simulink model "r_1f_2x_4a_r340"  (Simulink 7.2)
settings: Solver=ode45  StartTime=0.0  StopTime=1  RelTol=1e-3
undefined symbols (not defined in the file): ROACH
libraries: xbsIndex_r4 x2477, xps_library x100, casper_library x62, xbsReplacements_r4 x50
(part 3 of 20)

== system r_1f_2x_4a_r340/Subsystem4 ==
  led_up : Inport  OutDataType=sfix(16)  OutScaling=2^0
  led_rx : Inport  Port=2  OutDataType=sfix(16)  OutScaling=2^0
  data_in : Inport  Port=3  OutDataType=sfix(16)  OutScaling=2^0
  valid_in : Inport  Port=4  OutDataType=sfix(16)  OutScaling=2^0
  ip_in : Inport  Port=5  OutDataType=sfix(16)  OutScaling=2^0
  eof_in : Inport  Port=6  OutDataType=sfix(16)  OutScaling=2^0
  bad_frame : Inport  Port=7  OutDataType=sfix(16)  OutScaling=2^0
  overrun : Inport  Port=8  OutDataType=sfix(16)  OutScaling=2^0
  ack_in : Inport  Port=9  OutDataType=sfix(16)  OutScaling=2^0
  Concat1 : xbsIndex_r4/Concat  num_inputs=8  dbl_ovrd=off  block_type=concat
      settings: block_version=10.1.3
  Convert{1..6,18} (7 alike) : xbsIndex_r4/Convert  arith_type=Unsigned  n_bits=1  bin_pt=0  quantization=Truncate  overflow=Wrap  latency=0  dbl_ovrd=off  pipeline=off  block_type=convert
      settings: xl_area=[0, 0, 0, 0, 0, 0, 0]  block_version=10.1.3
  Delay{1..4} (4 alike) : xbsIndex_r4/Delay  en=off  latency=2  dbl_ovrd=off  reg_retiming=on  block_type=delay
      settings: xl_area=[0, 0, 0, 0, 0, 0, 0]
  Delay{5..22} (18 alike) : xbsIndex_r4/Delay  en=off  latency=1  dbl_ovrd=off  reg_retiming=on  block_type=delay
      settings: xl_area=[0, 0, 0, 0, 0, 0, 0]
  Logical4 : xbsIndex_r4/Logical  logical_function=AND  inputs=2  en=off  latency=0  precision=Full  arith_type=Unsigned  n_bits=8  bin_pt=2  align_bp=on  dbl_ovrd=off  block_type=logical
      settings: xl_area=[0, 0, 0, 0, 0, 0, 0]
  Slice11 : xbsIndex_r4/Slice  nbits=32-5  boolean_output=off  mode=Lower Bit Location + Width  bit1=0  base1=MSB of Input  bit0=0  base0=LSB of Input  dbl_ovrd=off  block_type=slice
      settings: infoedit="Extracts a given range of bits from each input sample and presents it at the output.  The output type is ordinarily unsigned with binary point at zero, but can be Boolean when the slice is one bit wide.<P><P>Hardware notes: In hardware this block costs nothing."  block_version=10.1.3
  data_out : Outport  OutDataType=sfix(16)  OutScaling=2^0
  oob_out : Outport  Port=2  OutDataType=sfix(16)  OutScaling=2^0
  trig_out : Outport  Port=3  OutDataType=sfix(16)  OutScaling=2^0
  we_out : Outport  Port=4  OutDataType=sfix(16)  OutScaling=2^0
  wires:
  Convert2/1 -> Concat1/6
  Convert1/1 -> Concat1/5
  Convert18/1 -> Concat1/4
  Convert4/1 -> Concat1/3
  Convert3/1 -> Concat1/2
  Slice11/1 -> Concat1/1
  Delay8/1 -> Delay17/1
  led_rx/1 -> Delay8/1
  Delay7/1 -> Delay16/1
  led_up/1 -> Delay7/1
  Delay11/1 -> Delay20/1
  overrun/1 -> Delay11/1
  Delay10/1 -> Delay19/1
  bad_frame/1 -> Delay10/1
  Delay13/1 -> Delay22/1
  Delay12/1 -> Delay21/1
  Delay9/1 -> Delay18/1
  Delay6/1 -> Delay15/1
  Delay5/1 -> Delay14/1
  Delay3/1 -> we_out/1
  Delay2/1 -> trig_out/1
  ack_in/1 -> Delay13/1
  eof_in/1 -> Delay9/1
  valid_in/1 -> Delay12/1
  Logical4/1 -> Delay2/1
  Delay4/1 -> oob_out/1
  Delay1/1 -> data_out/1
  ip_in/1 -> Delay6/1
  data_in/1 -> Delay5/1
  Concat1/1 -> Delay4/1
  Convert5/1 -> Concat1/7
  Convert6/1 -> Concat1/8
  Delay14/1 -> Delay1/1
  Delay15/1 -> Slice11/1
  Delay16/1 -> Convert3/1
  Delay17/1 -> Convert4/1
  Delay18/1 -> Convert18/1, Logical4/1
  Delay19/1 -> Convert1/1
  Delay20/1 -> Convert2/1
  Delay21/1 -> Convert5/1, Delay3/1, Logical4/2
  Delay22/1 -> Convert6/1

== system r_1f_2x_4a_r340/Subsystem5 ==
  rst : Inport  OutDataType=sfix(16)  OutScaling=2^0
  we : Inport  Port=2  OutDataType=sfix(16)  OutScaling=2^0
  eof : Inport  Port=3  OutDataType=sfix(16)  OutScaling=2^0
  flag : Inport  Port=4  OutDataType=sfix(16)  OutScaling=2^0
  Delay{1..3,40} (4 alike) : xbsIndex_r4/Delay  en=off  latency=2  dbl_ovrd=off  reg_retiming=on  block_type=delay
      settings: xl_area=[0, 0, 0, 0, 0, 0, 0]
  Logical2 : xbsIndex_r4/Logical  logical_function=AND  inputs=2  en=off  latency=0  precision=Full  arith_type=Unsigned  n_bits=8  bin_pt=2  align_bp=on  dbl_ovrd=off  block_type=logical
      settings: xl_area=[0, 0, 0, 0, 0, 0, 0]
  payload_cnt1 : xbsIndex_r4/Counter  cnt_type=Free Running  cnt_to=0  operation=Up  start_count=0  cnt_by_val=1  arith_type=Unsigned  n_bits=32  bin_pt=0  load_pin=off  rst=on  en=on  explicit_period=on  period=1  dbl_ovrd=off  use_behavioral_HDL=off  implementation=Fabric  use_rpm=on  block_type=counter
      settings: xl_area=[0, 0, 0, 0, 0, 0, 0]
  payload_cnt2 : xbsIndex_r4/Counter  cnt_type=Free Running  cnt_to=0  operation=Up  start_count=0  cnt_by_val=1  arith_type=Unsigned  n_bits=32  bin_pt=0  load_pin=off  rst=on  en=on  explicit_period=on  period=1  dbl_ovrd=off  use_behavioral_HDL=off  implementation=Fabric  use_rpm=on  block_type=counter
      settings: xl_area=[0, 0, 0, 0, 0, 0, 0]
  posedge : casper_library/Misc/posedge  Tag=Rising Edge Detector  SystemSampleTime=-1
  rx_cnt : Outport  OutDataType=sfix(16)  OutScaling=2^0
  err_cnt : Outport  Port=2  OutDataType=sfix(16)  OutScaling=2^0
  wires:
  Logical2/1 -> posedge/1
  posedge/1 -> payload_cnt1/2
  eof/1 -> Delay1/1
  rst/1 -> Delay2/1
  payload_cnt1/1 -> rx_cnt/1
  we/1 -> Delay40/1
  Delay40/1 -> Logical2/1
  Delay1/1 -> Logical2/2
  Delay2/1 -> payload_cnt1/1, payload_cnt2/1
  flag/1 -> Delay3/1
  payload_cnt2/1 -> err_cnt/1
  Delay3/1 -> payload_cnt2/2

== system r_1f_2x_4a_r340/Subsystem9 ==
  data_in : Inport  OutDataType=sfix(16)  OutScaling=2^0
  oob_in : Inport  Port=2  OutDataType=sfix(16)  OutScaling=2^0
  we_in : Inport  Port=3  OutDataType=sfix(16)  OutScaling=2^0
  link_down : Inport  Port=4  OutDataType=sfix(16)  OutScaling=2^0
  Concat2 : xbsIndex_r4/Concat  num_inputs=2  dbl_ovrd=off  block_type=concat
      settings: block_version=10.1.3
  Constant9 : xbsIndex_r4/Constant  arith_type=Boolean  const=1  n_bits=1  bin_pt=0  explicit_period=on  period=1  equ=P=C  opselect=C  inp2=PCIN>>17  opr=+  inp1=P  carry=CIN  dbl_ovrd=off  block_type=constant
  Convert : xbsIndex_r4/Convert  arith_type=Unsigned  n_bits=32  bin_pt=0  quantization=Truncate  overflow=Wrap  latency=0  dbl_ovrd=off  pipeline=off  block_type=convert
      settings: block_version=10.1.3
  Convert1 : xbsIndex_r4/Convert  arith_type=Unsigned  n_bits=1  bin_pt=0  quantization=Truncate  overflow=Wrap  latency=0  dbl_ovrd=off  pipeline=off  block_type=convert
      settings: block_version=10.1.3
  Delay{1..2,4,6} (4 alike) : xbsIndex_r4/Delay  en=off  latency=2  dbl_ovrd=off  reg_retiming=on  block_type=delay
      settings: xl_area=[0, 0, 0, 0, 0, 0, 0]
  Slice3 : xbsIndex_r4/Slice  nbits=1  boolean_output=on  mode=Lower Bit Location + Width  bit1=0  base1=MSB of Input  bit0=1  base0=LSB of Input  dbl_ovrd=off  block_type=slice
      settings: infoedit="Extracts a given range of bits from each input sample and presents it at the output.  The output type is ordinarily unsigned with binary point at zero, but can be Boolean when the slice is one bit wide.<P><P>Hardware notes: In hardware this block costs nothing."  block_version=10.1.3
  data_out : Outport  OutDataType=sfix(16)  OutScaling=2^0
  oob_out : Outport  Port=2  OutDataType=sfix(16)  OutScaling=2^0
  we_out : Outport  Port=3  OutDataType=sfix(16)  OutScaling=2^0
  trig_out : Outport  Port=4  OutDataType=sfix(16)  OutScaling=2^0
  stop : Outport  Port=5  OutDataType=sfix(16)  OutScaling=2^0
  wires:
  Delay1/1 -> data_out/1
  Delay4/1 -> Convert/1
  Delay6/1 -> trig_out/1
  oob_in/1 -> Concat2/2, Slice3/1
  Slice3/1 -> Delay6/1
  Delay2/1 -> we_out/1
  we_in/1 -> Delay2/1
  link_down/1 -> Convert1/1
  data_in/1 -> Delay1/1
  Concat2/1 -> Delay4/1
  Convert/1 -> oob_out/1
  Constant9/1 -> stop/1
  Convert1/1 -> Concat2/1

== system r_1f_2x_4a_r340/demux_gbe0 ==
  we : Inport  OutDataType=sfix(16)  OutScaling=2^0
  mcnt : Inport  Port=2  OutDataType=sfix(16)  OutScaling=2^0
  Constant1 : xbsIndex_r4/Constant  arith_type=Unsigned  const=nx  n_bits=share_bits  bin_pt=0  explicit_period=on  period=1  equ=P=C  opselect=C  inp2=PCIN>>17  opr=+  inp1=P  carry=CIN  dbl_ovrd=off  block_type=constant
  Logical3 : xbsIndex_r4/Logical  logical_function=AND  inputs=2  en=off  latency=0  precision=Full  arith_type=Unsigned  n_bits=8  bin_pt=2  align_bp=on  dbl_ovrd=off  block_type=logical
      settings: xl_area=[0, 0, 0, 0, 0, 0, 0]
  Relational : xbsIndex_r4/Relational  mode=a=b  en=off  latency=0  dbl_ovrd=off  block_type=relational
      settings: xl_area=[0, 0, 0, 0, 0, 0, 0]  block_version=10.1.3
  Slice4 : xbsIndex_r4/Slice  nbits=share_bits  boolean_output=off  mode=Lower Bit Location + Width  bit1=0  base1=MSB of Input  bit0=n_xeng_bits-share_bits  base0=LSB of Input  dbl_ovrd=off  block_type=slice
      settings: infoedit="Extracts a given range of bits from each input sample and presents it at the output.  The output type is ordinarily unsigned with binary point at zero, but can be Boolean when the slice is one bit wide.<P><P>Hardware notes: In hardware this block costs nothing."  block_version=10.1.3
  valid : Outport  OutDataType=sfix(16)  OutScaling=2^0
  wires:
  we/1 -> Logical3/1
  Logical3/1 -> valid/1
  mcnt/1 -> Slice4/1
  Slice4/1 -> Relational/1
  Constant1/1 -> Relational/2
  Relational/1 -> Logical3/2

== system r_1f_2x_4a_r340/demux_gbe1 ==
  we : Inport  OutDataType=sfix(16)  OutScaling=2^0
  mcnt : Inport  Port=2  OutDataType=sfix(16)  OutScaling=2^0
  Constant1 : xbsIndex_r4/Constant  arith_type=Unsigned  const=nx  n_bits=share_bits  bin_pt=0  explicit_period=on  period=1  equ=P=C  opselect=C  inp2=PCIN>>17  opr=+  inp1=P  carry=CIN  dbl_ovrd=off  block_type=constant
  Logical3 : xbsIndex_r4/Logical  logical_function=AND  inputs=2  en=off  latency=0  precision=Full  arith_type=Unsigned  n_bits=8  bin_pt=2  align_bp=on  dbl_ovrd=off  block_type=logical
      settings: xl_area=[0, 0, 0, 0, 0, 0, 0]
  Relational : xbsIndex_r4/Relational  mode=a=b  en=off  latency=0  dbl_ovrd=off  block_type=relational
      settings: xl_area=[0, 0, 0, 0, 0, 0, 0]  block_version=10.1.3
  Slice4 : xbsIndex_r4/Slice  nbits=share_bits  boolean_output=off  mode=Lower Bit Location + Width  bit1=0  base1=MSB of Input  bit0=n_xeng_bits-share_bits  base0=LSB of Input  dbl_ovrd=off  block_type=slice
      settings: infoedit="Extracts a given range of bits from each input sample and presents it at the output.  The output type is ordinarily unsigned with binary point at zero, but can be Boolean when the slice is one bit wide.<P><P>Hardware notes: In hardware this block costs nothing."  block_version=10.1.3
  valid : Outport  OutDataType=sfix(16)  OutScaling=2^0
  wires:
  Relational/1 -> Logical3/2
  Constant1/1 -> Relational/2
  Slice4/1 -> Relational/1
  mcnt/1 -> Slice4/1
  Logical3/1 -> valid/1
  we/1 -> Logical3/1

== system r_1f_2x_4a_r340/gbe_err ==
  rst : Inport  OutDataType=sfix(16)  OutScaling=2^0
  discard : Inport  Port=2  OutDataType=sfix(16)  OutScaling=2^0
  eof : Inport  Port=3  OutDataType=sfix(16)  OutScaling=2^0
  valid : Inport  Port=4  OutDataType=sfix(16)  OutScaling=2^0
  Constant2 : xbsIndex_r4/Constant  arith_type=Unsigned  const=packet_len-1  n_bits=packet_bits+1  bin_pt=0  explicit_period=on  period=1  equ=P=C  opselect=C  inp2=PCIN>>17  opr=+  inp1=P  carry=CIN  dbl_ovrd=off  block_type=constant
  Delay{1..3,17} (4 alike) : xbsIndex_r4/Delay  en=off  latency=2  dbl_ovrd=off  reg_retiming=on  block_type=delay
      settings: xl_area=[0, 0, 0, 0, 0, 0, 0]
  Inverter3 : xbsIndex_r4/Inverter  en=off  latency=0  dbl_ovrd=off  block_type=inv
      settings: xl_area=[0, 0, 0, 0, 0, 0, 0]
  Logical1 : xbsIndex_r4/Logical  logical_function=AND  inputs=2  en=off  latency=0  precision=Full  arith_type=Unsigned  n_bits=8  bin_pt=2  align_bp=on  dbl_ovrd=off  block_type=logical
      settings: xl_area=[0, 0, 0, 0, 0, 0, 0]
  Logical2 : xbsIndex_r4/Logical  logical_function=OR  inputs=2  en=off  latency=0  precision=Full  arith_type=Unsigned  n_bits=8  bin_pt=2  align_bp=on  dbl_ovrd=off  block_type=logical
      settings: xl_area=[0, 0, 0, 0, 0, 0, 0]
  Logical3 : xbsIndex_r4/Logical  logical_function=AND  inputs=3  en=off  latency=0  precision=Full  arith_type=Unsigned  n_bits=8  bin_pt=2  align_bp=on  dbl_ovrd=off  block_type=logical
      settings: xl_area=[0, 0, 0, 0, 0, 0, 0]
  Logical4 : xbsIndex_r4/Logical  logical_function=AND  inputs=2  en=off  latency=0  precision=Full  arith_type=Unsigned  n_bits=8  bin_pt=2  align_bp=on  dbl_ovrd=off  block_type=logical
      settings: xl_area=[0, 0, 0, 0, 0, 0, 0]
  Logical5 : xbsIndex_r4/Logical  logical_function=AND  inputs=2  en=off  latency=0  precision=Full  arith_type=Unsigned  n_bits=8  bin_pt=2  align_bp=on  dbl_ovrd=off  block_type=logical
      settings: xl_area=[0, 0, 0, 0, 0, 0, 0]
  Logical6 : xbsIndex_r4/Logical  logical_function=OR  inputs=2  en=off  latency=0  precision=Full  arith_type=Unsigned  n_bits=8  bin_pt=2  align_bp=on  dbl_ovrd=off  block_type=logical
      settings: xl_area=[0, 0, 0, 0, 0, 0, 0]
  Relational1 : xbsIndex_r4/Relational  mode=a!=b  en=off  latency=0  dbl_ovrd=off  block_type=relational
      settings: xl_area=[0, 0, 0, 0, 0, 0, 0]  block_version=10.1.3
  payload_cnt : xbsIndex_r4/Counter  cnt_type=Free Running  cnt_to=0  operation=Up  start_count=0  cnt_by_val=1  arith_type=Unsigned  n_bits=32  bin_pt=0  load_pin=off  rst=on  en=on  explicit_period=off  period=1  dbl_ovrd=off  use_behavioral_HDL=off  implementation=Fabric  use_rpm=on  block_type=counter
      settings: xl_area=[0, 0, 0, 0, 0, 0, 0]
  payload_cnt1 : xbsIndex_r4/Counter  cnt_type=Free Running  cnt_to=0  operation=Up  start_count=0  cnt_by_val=1  arith_type=Unsigned  n_bits=32  bin_pt=0  load_pin=off  rst=on  en=on  explicit_period=off  period=1  dbl_ovrd=off  use_behavioral_HDL=off  implementation=Fabric  use_rpm=on  block_type=counter
      settings: xl_area=[0, 0, 0, 0, 0, 0, 0]
  payload_cnt2 : xbsIndex_r4/Counter  cnt_type=Free Running  cnt_to=packet_len - 1  operation=Up  start_count=0  cnt_by_val=1  arith_type=Unsigned  n_bits=packet_bits+1  bin_pt=0  load_pin=off  rst=on  en=on  explicit_period=off  period=1  dbl_ovrd=off  use_behavioral_HDL=off  implementation=Fabric  use_rpm=on  block_type=counter
      settings: xl_area=[0, 0, 0, 0, 0, 0, 0]
  tx_cnt : Outport  OutDataType=sfix(16)  OutScaling=2^0
  err_cnt : Outport  Port=2  OutDataType=sfix(16)  OutScaling=2^0
  wires:
  Inverter3/1 -> Logical3/1
  Logical3/1 -> payload_cnt/2
  discard/1 -> Delay1/1
  eof/1 -> Delay2/1
  valid/1 -> Delay3/1
  rst/1 -> Delay17/1
  payload_cnt/1 -> tx_cnt/1
  payload_cnt1/1 -> err_cnt/1
  Delay17/1 -> payload_cnt/1, payload_cnt1/1
  Delay1/1 -> Inverter3/1, Logical4/2, Logical6/1
  Delay2/1 -> Logical3/2, Logical6/2
  Delay3/1 -> Logical3/3, Logical4/1, payload_cnt2/2, Logical1/2
  payload_cnt2/1 -> Relational1/1
  Constant2/1 -> Relational1/2
  Relational1/1 -> Logical5/2
  Logical1/1 -> payload_cnt2/1, Logical5/1
  Logical5/1 -> Logical2/2
  Logical2/1 -> payload_cnt1/2
  Logical4/1 -> Logical2/1
  Logical6/1 -> Logical1/1

== system r_1f_2x_4a_r340/loopback_fifo0 ==
  note: Only take packets transmitted
      | to my own IP address
  din : Inport  OutDataType=sfix(16)  OutScaling=2^0
  we : Inport  Port=2  OutDataType=sfix(16)  OutScaling=2^0
  dest_ip : Inport  Port=3  OutDataType=sfix(16)  OutScaling=2^0
  eof : Inport  Port=4  OutDataType=sfix(16)  OutScaling=2^0
  discard : Inport  Port=5  OutDataType=sfix(16)  OutScaling=2^0
  my_ip : Inport  Port=6  OutDataType=sfix(16)  OutScaling=2^0
  re : Inport  Port=7  OutDataType=sfix(16)  OutScaling=2^0
  Concat : xbsIndex_r4/Concat  num_inputs=2  dbl_ovrd=off  block_type=concat
      settings: block_version=10.1.3
  Constant1 : xbsIndex_r4/Constant  arith_type=Boolean  const=1  n_bits=2  bin_pt=0  explicit_period=on  period=1  equ=P=C  opselect=C  inp2=PCIN>>17  opr=+  inp1=P  carry=CIN  dbl_ovrd=off  block_type=constant
  Convert : xbsIndex_r4/Convert  arith_type=Unsigned  n_bits=1  bin_pt=0  quantization=Truncate  overflow=Wrap  latency=0  dbl_ovrd=off  pipeline=off  block_type=convert
      settings: xl_area=[0, 0, 0, 0, 0, 0, 0]  block_version=10.1.3
  Inverter1 : xbsIndex_r4/Inverter  en=off  latency=0  dbl_ovrd=off  block_type=inv
      settings: xl_area=[0, 0, 0, 0, 0, 0, 0]
  Logical1 : xbsIndex_r4/Logical  logical_function=OR  inputs=2  en=off  latency=0  precision=Full  arith_type=Unsigned  n_bits=8  bin_pt=2  align_bp=on  dbl_ovrd=off  block_type=logical
      settings: xl_area=[0, 0, 0, 0, 0, 0, 0]
  Logical2 : xbsIndex_r4/Logical  logical_function=AND  inputs=2  en=off  latency=0  precision=Full  arith_type=Unsigned  n_bits=8  bin_pt=2  align_bp=on  dbl_ovrd=off  block_type=logical
      settings: xl_area=[0, 0, 0, 0, 0, 0, 0]
  Logical4 : xbsIndex_r4/Logical  logical_function=AND  inputs=2  en=off  latency=0  precision=Full  arith_type=Unsigned  n_bits=8  bin_pt=2  align_bp=on  dbl_ovrd=off  block_type=logical
      settings: xl_area=[0, 0, 0, 0, 0, 0, 0]
  Relational1 : xbsIndex_r4/Relational  mode=a=b  en=off  latency=1  dbl_ovrd=off  block_type=relational
      settings: xl_area=[0, 0, 0, 0, 0, 0, 0]  block_version=10.1.3
  Slice : xbsIndex_r4/Slice  nbits=1  boolean_output=on  mode=Upper Bit Location + Width  bit1=0  base1=MSB of Input  bit0=0  base0=LSB of Input  dbl_ovrd=off  block_type=slice
      settings: infoedit="Extracts a given range of bits from each input sample and presents it at the output.  The output type is ordinarily unsigned with binary point at zero, but can be Boolean when the slice is one bit wide.<P><P>Hardware notes: In hardware this block costs nothing."  block_version=10.1.3
  Slice1 : xbsIndex_r4/Slice  nbits=64  boolean_output=off  mode=Lower Bit Location + Width  bit1=-1  base1=MSB of Input  bit0=0  base0=LSB of Input  dbl_ovrd=off  block_type=slice
      settings: infoedit="Extracts a given range of bits from each input sample and presents it at the output.  The output type is ordinarily unsigned with binary point at zero, but can be Boolean when the slice is one bit wide.<P><P>Hardware notes: In hardware this block costs nothing."  block_version=10.1.3
  Terminator{1..4} (4 alike) : Terminator
  fifo : Subsystem
  rollback_fifo : Subsystem  mask{depth_bits=8}
      mask prompts: depth_bits "Fifo Depth (2^?):"
      mask help: A rollback fifo behaves as a normal fifo, except that data which are written in have to be "committed" before they become available for readout. Moreover, any data which are written in and have not been committed can be "discarded", and will never be made available for readout.
  dout : Outport  OutDataType=sfix(16)  OutScaling=2^0
  valid : Outport  Port=2  OutDataType=sfix(16)  OutScaling=2^0
  ip_addr : Outport  Port=3  OutDataType=sfix(16)  OutScaling=2^0
  eof_out : Outport  Port=4  OutDataType=sfix(16)  OutScaling=2^0
  data_rdy : Outport  Port=5  OutDataType=sfix(16)  OutScaling=2^0
  overflow : Outport  Port=6  OutDataType=sfix(16)  OutScaling=2^0
  wires:
  Logical2/1 -> Logical1/1
  Inverter1/1 -> Logical2/2
  discard/1 -> Logical1/2
  Logical1/1 -> rollback_fifo/4(discard)
  Convert/1 -> Concat/1
  din/1 -> Concat/2
  Concat/1 -> rollback_fifo/1(din)
  Logical4/1 -> rollback_fifo/3(commit)
  we/1 -> rollback_fifo/2(we)
  eof/1 -> Logical2/1, Logical4/1, Convert/1
  Relational1/1 -> Inverter1/1, Logical4/2
  dest_ip/1 -> Relational1/1
  Constant1/1 -> rollback_fifo/5(re)
  rollback_fifo/2(valid) -> fifo/2(valid_in)
  re/1 -> fifo/4(re)
  fifo/2(valid) -> valid/1
  fifo/3(eof) -> eof_out/1
  fifo/1(data) -> dout/1
  fifo/4(data_rdy) -> data_rdy/1
  my_ip/1 -> Relational1/2, ip_addr/1
  Slice1/1 -> fifo/1(d_in)
  rollback_fifo/1(dout) -> Slice1/1, Slice/1
  Slice/1 -> fifo/3(eof_in)
  rollback_fifo/5(empty) -> Terminator2/1
  rollback_fifo/4(full) -> Terminator1/1
  rollback_fifo/3(wr_ack) -> Terminator3/1
  fifo/5(we) -> Terminator4/1
  fifo/6(full) -> overflow/1

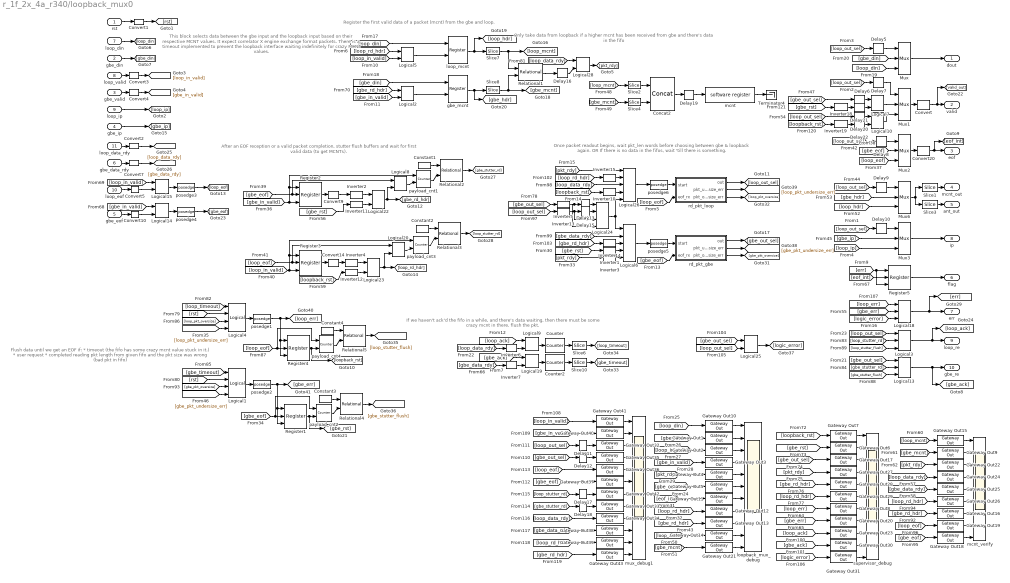
== system r_1f_2x_4a_r340/loopback_mux0 ==
  note: Once packet readout begins, wait pkt_len words
      | before choosing between gbe & loopback again.
      | OR
      | If there is no data in the fifos, wait 'till there is something.
  note: Only take data from loopback
      | if a higher mcnt has been
      | received from gbe and there's data
      | in the fifo
  note: This block selects data between the gbe input
      | and the loopback input based on their respective MCNT values.
      | It expect correlator X engine exchange format packets.
      |
      | There is a timeout implemented to prevent the loopback interface
      | waiting indefinitely for crazy MCNT values.
  note: Register the first valid
      | data of a packet (mcnt)
      | from the gbe and loop.
  note: After an EOF reception or a valid packet completion,
      |  stutter flush buffers and wait for first valid data (to get MCNTs).
  note: Flush data until we get an EOF if:
      |  * timeout (the fifo has some crazy mcnt value stuck in it.)
      |  * user request
      |  * completed reading pkt length from given fifo
      |     and the pkt size was wrong (bad pkt in fifo)
  note: If we haven't ack'd the fifo in a while,
      | and there's data waiting,
      | then there must be some crazy mcnt in there.
      | flush the pkt.
  rst : Inport  OutDataType=sfix(16)  OutScaling=2^0
  gbe_din : Inport  Port=2  OutDataType=sfix(16)  OutScaling=2^0
  gbe_valid : Inport  Port=3  OutDataType=sfix(16)  OutScaling=2^0
  gbe_ip : Inport  Port=4  OutDataType=sfix(16)  OutScaling=2^0
  gbe_eof : Inport  Port=5  OutDataType=sfix(16)  OutScaling=2^0
  gbe_data_rdy : Inport  Port=6  OutDataType=sfix(16)  OutScaling=2^0
  loop_din : Inport  Port=7  OutDataType=sfix(16)  OutScaling=2^0
  loop_valid : Inport  Port=8  OutDataType=sfix(16)  OutScaling=2^0
  loop_ip : Inport  Port=9  OutDataType=sfix(16)  OutScaling=2^0
  loop_eof : Inport  Port=10  OutDataType=sfix(16)  OutScaling=2^0
  loop_data_rdy : Inport  Port=11  OutDataType=sfix(16)  OutScaling=2^0
  Concat2 : xbsIndex_r4/Concat  num_inputs=2  dbl_ovrd=off  block_type=concat
      settings: block_version=10.1.3
  Constant{1..4} (4 alike) : xbsIndex_r4/Constant  arith_type=Unsigned  const=1  n_bits=ceil(log2(fifo_latency))  bin_pt=0  explicit_period=on  period=1  equ=P=C  opselect=C  inp2=PCIN>>17  opr=+  inp1=P  carry=CIN  dbl_ovrd=off  block_type=constant
  Convert{,1,9,14,20} (5 alike) : xbsIndex_r4/Convert  arith_type=Boolean  n_bits=8  bin_pt=6  quantization=Truncate  overflow=Wrap  latency=0  dbl_ovrd=off  pipeline=off  block_type=convert
      settings: xl_area=[0, 0, 0, 0, 0, 0, 0]  block_version=10.1.3
  Convert{2..5,7,10} (6 alike) : xbsIndex_r4/Convert  arith_type=Boolean  n_bits=1  bin_pt=0  quantization=Truncate  overflow=Wrap  latency=0  dbl_ovrd=off  pipeline=off  block_type=convert
      settings: xl_area=[0, 0, 0, 0, 0, 0, 0]  block_version=10.1.3
  Counter : xbsIndex_r4/Counter  cnt_type=Free Running  cnt_to=Inf  operation=Up  start_count=0  cnt_by_val=1  arith_type=Unsigned  n_bits=timeout_bits+1  bin_pt=0  load_pin=off  rst=on  en=off  explicit_period=off  period=1  dbl_ovrd=off  use_behavioral_HDL=off  implementation=Fabric  use_rpm=on  block_type=counter
      settings: xl_area=[0, 0, 0, 0, 0, 0, 0]
  Counter2 : xbsIndex_r4/Counter  cnt_type=Free Running  cnt_to=Inf  operation=Up  start_count=0  cnt_by_val=1  arith_type=Unsigned  n_bits=timeout_bits+1  bin_pt=0  load_pin=off  rst=on  en=off  explicit_period=off  period=1  dbl_ovrd=off  use_behavioral_HDL=off  implementation=Fabric  use_rpm=on  block_type=counter
      settings: xl_area=[0, 0, 0, 0, 0, 0, 0]
  Delay{5..13,15,17..18,20..22} (15 alike) : xbsIndex_r4/Delay  en=off  latency=fifo_latency  dbl_ovrd=off  reg_retiming=on  block_type=delay
      settings: xl_area=[0, 0, 0, 0, 0, 0, 0]
  Delay16 : xbsIndex_r4/Delay  en=off  latency=1  dbl_ovrd=off  reg_retiming=on  block_type=delay
      settings: xl_area=[0, 0, 0, 0, 0, 0, 0]
  Delay19 : xbsIndex_r4/Delay  en=off  latency=2  dbl_ovrd=off  reg_retiming=on  block_type=delay
      settings: xl_area=[0, 0, 0, 0, 0, 0, 0]
  Gateway Out{1..43} (43 alike) : xbsIndex_r4/Gateway Out  hdl_port=off  timing_constraint=None  locs_specified=off  LOCs={}  block_type=gatewayout
      settings: infoedit="Gateway out block.  Converts Xilinx fixed point inputs into ouputs of type Simulink integer, double, or fixed point.<P><P>Hardware notes:  In hardware these blocks become top level output ports or are discarded, depending on how they are configured."  xl_area=[0, 0, 0, 0, 0, 0, 0]  block_version=10.1.3
  Inverter{1..7,10..15,18..19} (15 alike) : xbsIndex_r4/Inverter  en=off  latency=0  dbl_ovrd=off  block_type=inv
      settings: xl_area=[0, 0, 0, 0, 0, 0, 0]
  Logical1 : xbsIndex_r4/Logical  logical_function=OR  inputs=4  en=off  latency=0  precision=Full  arith_type=Unsigned  n_bits=8  bin_pt=2  align_bp=on  dbl_ovrd=off  block_type=logical
      settings: xl_area=[0, 0, 0, 0, 0, 0, 0]
  Logical{2,5,7,10,14..15,22..23,25,28} (10 alike) : xbsIndex_r4/Logical  logical_function=AND  inputs=2  en=off  latency=0  precision=Full  arith_type=Unsigned  n_bits=8  bin_pt=2  align_bp=on  dbl_ovrd=off  block_type=logical
      settings: xl_area=[0, 0, 0, 0, 0, 0, 0]
  Logical13 : xbsIndex_r4/Logical  logical_function=OR  inputs=3  en=off  latency=0  precision=Full  arith_type=Unsigned  n_bits=8  bin_pt=2  align_bp=on  dbl_ovrd=off  block_type=logical
      settings: xl_area=[0, 0, 0, 0, 0, 0, 0]
  Logical18 : xbsIndex_r4/Logical  logical_function=OR  inputs=3  en=off  latency=1  precision=Full  arith_type=Unsigned  n_bits=8  bin_pt=2  align_bp=on  dbl_ovrd=off  block_type=logical
      settings: xl_area=[0, 0, 0, 0, 0, 0, 0]
  Logical{8..9,19..20} (4 alike) : xbsIndex_r4/Logical  logical_function=OR  inputs=2  en=off  latency=0  precision=Full  arith_type=Unsigned  n_bits=8  bin_pt=2  align_bp=on  dbl_ovrd=off  block_type=logical
      settings: xl_area=[0, 0, 0, 0, 0, 0, 0]
  Logical24 : xbsIndex_r4/Logical  logical_function=AND  inputs=4  en=off  latency=0  precision=Full  arith_type=Unsigned  n_bits=8  bin_pt=2  align_bp=on  dbl_ovrd=off  block_type=logical
      settings: xl_area=[0, 0, 0, 0, 0, 0, 0]
  Logical26 : xbsIndex_r4/Logical  logical_function=AND  inputs=5  en=off  latency=0  precision=Full  arith_type=Unsigned  n_bits=8  bin_pt=2  align_bp=on  dbl_ovrd=off  block_type=logical
      settings: xl_area=[0, 0, 0, 0, 0, 0, 0]
  Logical3 : xbsIndex_r4/Logical  logical_function=OR  inputs=3  en=off  latency=0  precision=Full  arith_type=Unsigned  n_bits=8  bin_pt=2  align_bp=on  dbl_ovrd=off  block_type=logical
      settings: xl_area=[0, 0, 0, 0, 0, 0, 0]
  Logical4 : xbsIndex_r4/Logical  logical_function=OR  inputs=4  en=off  latency=0  precision=Full  arith_type=Unsigned  n_bits=8  bin_pt=2  align_bp=on  dbl_ovrd=off  block_type=logical
      settings: xl_area=[0, 0, 0, 0, 0, 0, 0]
  Logical6 : xbsIndex_r4/Logical  logical_function=AND  inputs=5  en=off  latency=0  precision=Full  arith_type=Unsigned  n_bits=8  bin_pt=2  align_bp=on  dbl_ovrd=off  block_type=logical
      settings: xl_area=[0, 0, 0, 0, 0, 0, 0]
  Mux{,1..3,6} (5 alike) : xbsIndex_r4/Mux  inputs=2  en=off  latency=0  precision=Full  arith_type="Signed  (2's comp)"  n_bits=8  bin_pt=2  quantization=Truncate  overflow=Wrap  dbl_ovrd=off  block_type=mux
      settings: xl_area=[0, 0, 0, 0, 0, 0, 0]
  Register{1..5} (5 alike) : xbsIndex_r4/Register  init=0  rst=on  en=on  dbl_ovrd=off  block_type=register
      settings: xl_area=[0, 0, 0, 0, 0, 0, 0]
  Relational1 : xbsIndex_r4/Relational  mode=a<b  en=off  latency=1  dbl_ovrd=off  block_type=relational
      settings: xl_area=[0, 0, 0, 0, 0, 0, 0]  block_version=10.1.3
  Relational{2..5} (4 alike) : xbsIndex_r4/Relational  mode=a=b  en=off  latency=0  dbl_ovrd=off  block_type=relational
      settings: xl_area=[0, 0, 0, 0, 0, 0, 0]  block_version=10.1.3
  Slice1 : xbsIndex_r4/Slice  nbits=48  boolean_output=off  mode=Lower Bit Location + Width  bit1=0  base1=MSB of Input  bit0=16  base0=LSB of Input  dbl_ovrd=off  block_type=slice
      settings: infoedit="Extracts a given range of bits from each input sample and presents it at the output.  The output type is ordinarily unsigned with binary point at zero, but can be Boolean when the slice is one bit wide.<P><P>Hardware notes: In hardware this block costs nothing."  block_version=10.1.3
  Slice10 : xbsIndex_r4/Slice  nbits=1  boolean_output=on  mode=Upper Bit Location + Width  bit1=0  base1=MSB of Input  bit0=1  base0=LSB of Input  dbl_ovrd=off  block_type=slice
      settings: infoedit="Extracts a given range of bits from each input sample and presents it at the output.  The output type is ordinarily unsigned with binary point at zero, but can be Boolean when the slice is one bit wide.<P><P>Hardware notes: In hardware this block costs nothing."  block_version=10.1.3
  Slice2 : xbsIndex_r4/Slice  nbits=16  boolean_output=off  mode=Lower Bit Location + Width  bit1=0  base1=MSB of Input  bit0=0  base0=LSB of Input  dbl_ovrd=off  block_type=slice
      settings: infoedit="Extracts a given range of bits from each input sample and presents it at the output.  The output type is ordinarily unsigned with binary point at zero, but can be Boolean when the slice is one bit wide.<P><P>Hardware notes: In hardware this block costs nothing."  block_version=10.1.3
  Slice3 : xbsIndex_r4/Slice  nbits=16  boolean_output=off  mode=Lower Bit Location + Width  bit1=0  base1=MSB of Input  bit0=0  base0=LSB of Input  dbl_ovrd=off  block_type=slice
      settings: infoedit="Extracts a given range of bits from each input sample and presents it at the output.  The output type is ordinarily unsigned with binary point at zero, but can be Boolean when the slice is one bit wide.<P><P>Hardware notes: In hardware this block costs nothing."  block_version=10.1.3
  Slice4 : xbsIndex_r4/Slice  nbits=16  boolean_output=off  mode=Lower Bit Location + Width  bit1=0  base1=MSB of Input  bit0=0  base0=LSB of Input  dbl_ovrd=off  block_type=slice
      settings: infoedit="Extracts a given range of bits from each input sample and presents it at the output.  The output type is ordinarily unsigned with binary point at zero, but can be Boolean when the slice is one bit wide.<P><P>Hardware notes: In hardware this block costs nothing."  block_version=10.1.3
  Slice6 : xbsIndex_r4/Slice  nbits=1  boolean_output=on  mode=Upper Bit Location + Width  bit1=0  base1=MSB of Input  bit0=1  base0=LSB of Input  dbl_ovrd=off  block_type=slice
      settings: infoedit="Extracts a given range of bits from each input sample and presents it at the output.  The output type is ordinarily unsigned with binary point at zero, but can be Boolean when the slice is one bit wide.<P><P>Hardware notes: In hardware this block costs nothing."  block_version=10.1.3
  Slice7 : xbsIndex_r4/Slice  nbits=48  boolean_output=off  mode=Upper Bit Location + Width  bit1=0  base1=MSB of Input  bit0=0  base0=LSB of Input  dbl_ovrd=off  block_type=slice
      settings: infoedit="Extracts a given range of bits from each input sample and presents it at the output.  The output type is ordinarily unsigned with binary point at zero, but can be Boolean when the slice is one bit wide.<P><P>Hardware notes: In hardware this block costs nothing."  block_version=10.1.3
  Slice8 : xbsIndex_r4/Slice  nbits=48  boolean_output=off  mode=Upper Bit Location + Width  bit1=0  base1=MSB of Input  bit0=0  base0=LSB of Input  dbl_ovrd=off  block_type=slice
      settings: infoedit="Extracts a given range of bits from each input sample and presents it at the output.  The output type is ordinarily unsigned with binary point at zero, but can be Boolean when the slice is one bit wide.<P><P>Hardware notes: In hardware this block costs nothing."  block_version=10.1.3
  Terminator4 : Terminator
  gbe_mcnt : xbsIndex_r4/Register  init=0  rst=off  en=on  dbl_ovrd=off  block_type=register
      settings: xl_area=[0, 0, 0, 0, 0, 0, 0]
  loop_mcnt : xbsIndex_r4/Register  init=0  rst=off  en=on  dbl_ovrd=off  block_type=register
      settings: xl_area=[0, 0, 0, 0, 0, 0, 0]
  loopback_mux_debug : Scope  NumInputPorts=11
      settings: YMin=300~190.95~0~0.95~0.95~-1~-1~-1~-5~-5~-5  YMax=525~211.05~1~1.05~1.05~1~1~1~5~5~5  SaveName=ScopeData7
  mcnt : xps_library/software register  Tag=xps:sw_reg  SystemSampleTime=-1  io_dir=To Processor  arith_type=Unsigned  bitwidth=32  bin_pt=0  sample_period=1  gw_name=r_1f_2x_4a_r339_loopback_mux0_mcnt_user_data_in
      link override convert: arith_type=Unsigned  n_bits=32  bin_pt=0
  mcnt_verify : Scope  NumInputPorts=9
      settings: YMin=300~190.95~0~-5~-5~-5~-5~-5~-5  YMax=525~211.05~1~5~5~5~5~5~5  SaveName=ScopeData2
  mux_debug1 : Scope  NumInputPorts=12
      settings: YMin=300~190.95~0~-5~-5~-5~-5~-5~-5~-5~-5~-5  YMax=525~211.05~1~5~5~5~5~5~5~5~5~5  SaveName=ScopeData3
  payload_cnt{1..4} (4 alike) : xbsIndex_r4/Counter  cnt_type=Count Limited  cnt_to=max((fifo_latency + 1),2)  operation=Up  start_count=0  cnt_by_val=1  arith_type=Unsigned  n_bits=ceil(log2(fifo_latency))+1  bin_pt=0  load_pin=off  rst=on  en=on  explicit_period=on  period=1  dbl_ovrd=off  use_behavioral_HDL=off  implementation=Fabric  use_rpm=on  block_type=counter
      settings: xl_area=[0, 0, 0, 0, 0, 0, 0]
  posedge{1..6} (6 alike) : casper_library/Misc/posedge  Tag=Rising Edge Detector  SystemSampleTime=-1
  rd_pkt_gbe : Subsystem
  rd_pkt_loop : Subsystem
  supervisor_debug : Scope  NumInputPorts=11
      settings: YMin=300~190.95~0~-5~-5~-5~-5~-5~-5~-5~-5  YMax=525~211.05~1~5~5~5~5~5~5~5~5  SaveName=ScopeData18
  dout : Outport  OutDataType=sfix(16)  OutScaling=2^0
  valid : Outport  Port=2  OutDataType=sfix(16)  OutScaling=2^0
  eof : Outport  Port=3  OutDataType=sfix(16)  OutScaling=2^0
  mcnt_out : Outport  Port=4  OutDataType=sfix(16)  OutScaling=2^0
  ant_out : Outport  Port=5  OutDataType=sfix(16)  OutScaling=2^0
  flag : Outport  Port=6  OutDataType=sfix(16)  OutScaling=2^0
  err : Outport  Port=7  OutDataType=sfix(16)  OutScaling=2^0
  ip : Outport  Port=8  OutDataType=sfix(16)  OutScaling=2^0
  loop_re : Outport  Port=9  OutDataType=sfix(16)  OutScaling=2^0
  gbe_re : Outport  Port=10  OutDataType=sfix(16)  OutScaling=2^0
  wires:
  Mux/1 -> dout/1
  Mux1/1 -> Convert/1
  Mux2/1 -> Convert20/1
  gbe_valid/1 -> Convert4/1
  loop_valid/1 -> Convert3/1
  Convert/1 -> valid/1, [valid_out]
  Slice2/1 -> Concat2/1
  Slice4/1 -> Concat2/2
  Concat2/1 -> Delay19/1
  Delay5/1 -> Mux/1
  [loop_out_sel] -> Delay5/1
  [loop_out_sel] -> Delay8/1
  Gateway Out2/1 -> loopback_mux_debug/3
  Gateway Out3/1 -> loopback_mux_debug/4
  Gateway Out4/1 -> loopback_mux_debug/5
  Gateway Out5/1 -> loopback_mux_debug/6
  Convert3/1 -> [loop_in_valid]
  Convert4/1 -> [gbe_in_valid]
  Relational1/1 -> Delay16/1
  loop_din/1 -> [loop_din]
  gbe_din/1 -> [gbe_din]
  [gbe_din] -> Gateway Out1/1
  [loop_din] -> Gateway Out10/1
  [loop_in_valid] -> Gateway Out2/1
  [gbe_in_valid] -> Gateway Out3/1
  [gbe_out_sel] -> Gateway Out5/1
  [pkt_rdy] -> Gateway Out4/1
  Gateway Out11/1 -> loopback_mux_debug/7
  [eof_int] -> Gateway Out11/1
  [loop_rd_hdr] -> Gateway Out12/1
  Gateway Out12/1 -> loopback_mux_debug/8
  loop_eof/1 -> Convert5/1
  gbe_eof/1 -> Convert10/1
  Gateway Out13/1 -> loopback_mux_debug/9
  [gbe_rd_hdr] -> Gateway Out13/1
  [loop_out_sel] -> Delay7/1
  loop_ip/1 -> [loop_ip]
  gbe_ip/1 -> [gbe_ip]
  Mux3/1 -> ip/1
  [loop_out_sel] -> Delay10/1
  [loop_mcnt] -> Slice2/1
  [gbe_mcnt] -> Slice4/1
  Gateway Out10/1 -> loopback_mux_debug/1
  Gateway Out1/1 -> loopback_mux_debug/2
  Gateway Out14/1 -> loopback_mux_debug/10
  Gateway Out21/1 -> loopback_mux_debug/11
  [loop_mcnt] -> Gateway Out14/1
  [gbe_mcnt] -> Gateway Out21/1
  Gateway Out22/1 -> mcnt_verify/3
  Gateway Out15/1 -> mcnt_verify/1
  Gateway Out9/1 -> mcnt_verify/2
  Gateway Out24/1 -> mcnt_verify/4
  Gateway Out25/1 -> mcnt_verify/5
  Gateway Out26/1 -> mcnt_verify/6
  Gateway Out16/1 -> mcnt_verify/7
  [loop_mcnt] -> Gateway Out15/1
  [gbe_mcnt] -> Gateway Out9/1
  [pkt_rdy] -> Gateway Out22/1
  Slice7/1 -> Relational1/1, [loop_mcnt]
  Slice8/1 -> Relational1/2, [gbe_mcnt]
  Slice1/1 -> mcnt_out/1
  Slice3/1 -> ant_out/1
  Mux6/1 -> Slice1/1, Slice3/1
  Counter2/1 -> Slice10/1
  Logical1/1 -> posedge2/1
  Logical14/1 -> posedge4/1
  Logical15/1 -> posedge3/1
  Convert5/1 -> Logical15/2
  Convert10/1 -> Logical14/2
  [gbe_in_valid] -> Logical14/1
  [loop_in_valid] -> Logical15/1
  Gateway Out17/1 -> supervisor_debug/3
  Gateway Out7/1 -> supervisor_debug/1
  Gateway Out6/1 -> supervisor_debug/2
  Gateway Out27/1 -> supervisor_debug/4
  Gateway Out28/1 -> supervisor_debug/5
  Gateway Out29/1 -> supervisor_debug/6
  Gateway Out8/1 -> supervisor_debug/7
  [loopback_rst] -> Gateway Out7/1
  [gbe_rst] -> Gateway Out6/1
  [gbe_out_sel] -> Gateway Out17/1
  [pkt_rdy] -> Gateway Out27/1
  [gbe_rd_hdr] -> Gateway Out28/1
  [loop_rd_hdr] -> Gateway Out29/1
  Logical3/1 -> loop_re/1, [loop_ack]
  Logical13/1 -> gbe_re/1, [gbe_ack]
  rst/1 -> Convert1/1
  Logical18/1 -> err/1, [err]
  Counter/1 -> Slice6/1
  Logical4/1 -> posedge1/1
  Register2/1 -> Convert9/1
  Logical22/1 -> [gbe_rd_hdr], Logical8/2
  Register3/1 -> Convert14/1
  Convert14/1 -> Inverter4/1
  gbe_mcnt/1 -> Slice8/1, [gbe_hdr]
  loop_mcnt/1 -> Slice7/1, [loop_hdr]
  Logical5/1 -> loop_mcnt/2
  [loop_rd_hdr] -> Logical5/1
  [gbe_rd_hdr] -> Logical2/1
  [loop_in_valid] -> Logical5/2
  [gbe_in_valid] -> Logical2/2
  [loop_din] -> loop_mcnt/1
  [gbe_din] -> gbe_mcnt/1
  Convert1/1 -> [rst]
  Convert20/1 -> [eof_int], eof/1
  posedge1/1 -> Register4/3, Register4/1, [loop_err]
  posedge2/1 -> Register1/1, Register1/3, [gbe_err]
  posedge3/1 -> [loop_eof]
  posedge4/1 -> [gbe_eof]
  Logical2/1 -> gbe_mcnt/2
  [loop_out_sel] -> Delay20/1
  Delay19/1 -> mcnt/1
  mcnt/1 -> Terminator4/1
  gbe_data_rdy/1 -> Convert7/1
  loop_data_rdy/1 -> Convert2/1
  Convert2/1 -> [loop_data_rdy]
  Convert7/1 -> [gbe_data_rdy]
  payload_cnt1/1 -> Relational2/2
  Constant1/1 -> Relational2/1
  payload_cnt3/1 -> Relational3/2
  Constant2/1 -> Relational3/1
  [gbe_out_sel] -> Delay6/1
  posedge6/1 -> rd_pkt_loop/1(start)
  rd_pkt_loop/1(out) -> [loop_out_sel]
  rd_pkt_gbe/1(out) -> [gbe_out_sel]
  [gbe_in_valid] -> Register2/3, Register2/1, payload_cnt1/1
  [loop_in_valid] -> Register3/3, Register3/1, payload_cnt3/1
  [loop_eof] -> Register3/2, Logical20/1
  [gbe_eof] -> Register2/2, Logical8/1
  Logical8/1 -> payload_cnt1/2
  Logical20/1 -> payload_cnt3/2
  Relational2/1 -> [gbe_stutter_rd]
  Relational3/1 -> [loop_stutter_rd]
  rd_pkt_gbe/3(pkt_oversize_err) -> [gbe_pkt_oversize]
  Slice6/1 -> [loop_timeout]
  Slice10/1 -> [gbe_timeout]
  [rst] -> Logical1/2
  [rst] -> Logical4/2
  [gbe_timeout] -> Logical1/1
  [loop_timeout] -> Logical4/1
  [gbe_pkt_oversize] -> Logical1/3
  [loop_pkt_oversize] -> Logical4/3
  [gbe_eof] -> Register1/2, payload_cnt2/1
  payload_cnt2/1 -> Relational4/2
  Constant3/1 -> Relational4/1
  Relational4/1 -> [gbe_stutter_flush]
  Register1/1 -> payload_cnt2/2, [gbe_rst]
  [loop_eof] -> payload_cnt4/1, Register4/2
  payload_cnt4/1 -> Relational5/2
  Constant4/1 -> Relational5/1
  Register4/1 -> payload_cnt4/2, [loopback_rst]
  Relational5/1 -> [loop_stutter_flush]
  [gbe_out_sel] -> Logical13/1
  [gbe_stutter_rd] -> Logical13/2
  [gbe_stutter_flush] -> Logical13/3
  [loop_out_sel] -> Logical3/1
  [loop_stutter_rd] -> Logical3/2
  [loop_stutter_flush] -> Logical3/3
  Inverter2/1 -> Logical22/1
  Convert9/1 -> Inverter2/1
  [gbe_rst] -> Inverter11/1
  Inverter11/1 -> Logical22/2
  Inverter12/1 -> Logical23/2
  Logical23/1 -> Logical20/2, [loop_rd_hdr]
  Inverter4/1 -> Logical23/1
  [loopback_rst] -> Inverter12/1
  Gateway Out19/1 -> mcnt_verify/8
  Gateway Out18/1 -> mcnt_verify/9
  [loop_data_rdy] -> Gateway Out24/1
  [gbe_data_rdy] -> Gateway Out25/1
  [loop_rd_hdr] -> Gateway Out26/1
  [gbe_rd_hdr] -> Gateway Out16/1
  [loop_eof] -> Gateway Out19/1
  [gbe_eof] -> Gateway Out18/1
  Gateway Out20/1 -> supervisor_debug/8
  Gateway Out23/1 -> supervisor_debug/9
  [loop_err] -> Gateway Out8/1
  [gbe_err] -> Gateway Out20/1
  Gateway Out30/1 -> supervisor_debug/10
  [gbe_out_sel] -> Inverter5/1
  [loop_out_sel] -> Inverter13/1
  Inverter5/1 -> Delay13/1, Logical24/4
  Inverter13/1 -> Delay15/1, Logical24/3
  [loop_ack] -> Gateway Out23/1
  [gbe_ack] -> Gateway Out30/1
  [loop_rd_hdr] -> Inverter15/1
  [gbe_rd_hdr] -> Inverter14/1
  Delay8/1 -> Mux2/1
  Delay9/1 -> Mux6/1
  [loop_out_sel] -> Delay9/1
  Delay10/1 -> Mux3/1
  Delay7/1 -> Mux1/1
  [gbe_out_sel] -> Logical25/1
  [loop_out_sel] -> Logical25/2
  Logical25/1 -> [logic_error]
  Gateway Out31/1 -> supervisor_debug/11
  [logic_error] -> Gateway Out31/1
  Gateway Out32/1 -> mux_debug1/3
  Gateway Out41/1 -> mux_debug1/1
  Gateway Out40/1 -> mux_debug1/2
  Gateway Out35/1 -> mux_debug1/4
  Gateway Out36/1 -> mux_debug1/5
  Gateway Out37/1 -> mux_debug1/6
  Gateway Out42/1 -> mux_debug1/7
  Gateway Out33/1 -> mux_debug1/8
  Gateway Out34/1 -> mux_debug1/9
  Gateway Out38/1 -> mux_debug1/10
  Gateway Out39/1 -> mux_debug1/11
  [loop_in_valid] -> Gateway Out41/1
  [gbe_in_valid] -> Gateway Out40/1
  [loop_eof] -> Gateway Out36/1
  [gbe_eof] -> Gateway Out37/1
  [gbe_out_sel] -> Delay12/1
  [loop_out_sel] -> Delay11/1
  [loop_stutter_rd] -> Delay17/1
  [gbe_stutter_rd] -> Delay18/1
  Delay11/1 -> Gateway Out32/1
  Delay12/1 -> Gateway Out35/1
  Delay17/1 -> Gateway Out42/1
  Delay18/1 -> Gateway Out33/1
  [loop_data_rdy] -> Gateway Out34/1
  [gbe_data_rdy] -> Gateway Out38/1
  Gateway Out43/1 -> mux_debug1/12
  [loop_rd_hdr] -> Gateway Out39/1
  [gbe_rd_hdr] -> Gateway Out43/1
  Delay13/1 -> Logical24/1
  Delay15/1 -> Logical24/2
  [loopback_rst] -> Inverter10/1
  [gbe_rst] -> Inverter1/1
  [gbe_eof] -> rd_pkt_gbe/2(eof_rx)
  [gbe_din] -> Mux/2
  [loop_din] -> Mux/3
  Logical10/1 -> Mux1/3
  Logical7/1 -> Mux1/2
  [gbe_eof] -> Mux2/2
  [loop_eof] -> Mux2/3
  [gbe_hdr] -> Mux6/2
  [loop_hdr] -> Mux6/3
  [gbe_ip] -> Mux3/2
  [loop_ip] -> Mux3/3
  Delay6/1 -> Logical7/1
  rd_pkt_gbe/2(pkt_undersize_err) -> [gbe_pkt_undersize_err]
  rd_pkt_loop/2(pkt_undersize_err) -> [loop_pkt_undersize_err]
  [loop_err] -> Logical18/1
  [gbe_err] -> Logical18/2
  Logical9/1 -> Counter/1
  [loop_ack] -> Logical9/1
  [loop_data_rdy] -> Inverter6/1
  Inverter6/1 -> Logical9/2
  Inverter7/1 -> Logical19/2
  Logical19/1 -> Counter2/1
  [gbe_ack] -> Logical19/1
  [gbe_data_rdy] -> Inverter7/1
  Logical6/1 -> posedge5/1
  [loop_data_rdy] -> Logical26/3
  [gbe_data_rdy] -> Logical6/2
  [loop_data_rdy] -> Logical28/1
  Delay16/1 -> Logical28/2
  Logical28/1 -> [pkt_rdy]
  Inverter14/1 -> Logical6/3
  Inverter10/1 -> Logical26/4
  Inverter1/1 -> Logical6/4
  Inverter15/1 -> Logical26/2
  posedge5/1 -> rd_pkt_gbe/1(start)
  [pkt_rdy] -> Inverter3/1
  [loopback_rst] -> Inverter19/1
  [gbe_rst] -> Inverter18/1
  Inverter18/1 -> Delay21/1
  Inverter19/1 -> Delay22/1
  [loop_pkt_undersize_err] -> Logical4/4
  [gbe_pkt_undersize_err] -> Logical1/4
  Delay21/1 -> Logical7/2
  [loop_eof] -> rd_pkt_loop/2(eof_rx)
  rd_pkt_loop/3(pkt_oversize_err) -> [loop_pkt_oversize]
  [logic_error] -> Logical18/3
  Logical24/1 -> Logical6/1, Logical26/5
  Delay20/1 -> Logical10/1
  Delay22/1 -> Logical10/2
  [pkt_rdy] -> Logical26/1
  Inverter3/1 -> Logical6/5
  Logical26/1 -> posedge6/1
  [err] -> Register5/1, Register5/3
  [eof_int] -> Register5/2
  Register5/1 -> flag/1

== system r_1f_2x_4a_r340/miss_cnt0 ==
  rst : Inport  OutDataType=sfix(16)  OutScaling=2^0
  valid : Inport  Port=2  OutDataType=sfix(16)  OutScaling=2^0
  rcvd : Inport  Port=3  OutDataType=sfix(16)  OutScaling=2^0
  sync : Inport  Port=4  OutDataType=sfix(16)  OutScaling=2^0
  Counter1 : xbsIndex_r4/Counter  cnt_type=Free Running  cnt_to=1023  operation=Up  start_count=0  cnt_by_val=1  arith_type=Unsigned  n_bits=32  bin_pt=0  load_pin=off  rst=on  en=on  explicit_period=on  period=1  dbl_ovrd=off  use_behavioral_HDL=off  implementation=Fabric  use_rpm=on  block_type=counter
      settings: xl_area=[0, 0, 0, 0, 0, 0, 0]
  Counter3 : xbsIndex_r4/Counter  cnt_type=Free Running  cnt_to=1023  operation=Up  start_count=0  cnt_by_val=1  arith_type=Unsigned  n_bits=32  bin_pt=0  load_pin=off  rst=on  en=on  explicit_period=on  period=1  dbl_ovrd=off  use_behavioral_HDL=off  implementation=Fabric  use_rpm=on  block_type=counter
      settings: xl_area=[0, 0, 0, 0, 0, 0, 0]
  Delay{1..3,55} (4 alike) : xbsIndex_r4/Delay  en=off  latency=2  dbl_ovrd=off  reg_retiming=on  block_type=delay
      settings: xl_area=[0, 0, 0, 0, 0, 0, 0]
  Inverter : xbsIndex_r4/Inverter  en=off  latency=0  dbl_ovrd=off  block_type=inv
      settings: xl_area=[0, 0, 0, 0, 0, 0, 0]
  Logical2 : xbsIndex_r4/Logical  logical_function=AND  inputs=2  en=off  latency=0  precision=Full  arith_type=Unsigned  n_bits=8  bin_pt=2  align_bp=on  dbl_ovrd=off  block_type=logical
      settings: xl_area=[0, 0, 0, 0, 0, 0, 0]
  Register : xbsIndex_r4/Register  init=0  rst=off  en=on  dbl_ovrd=off  block_type=register
  Slice : xbsIndex_r4/Slice  nbits=16  boolean_output=off  mode=Two Bit Locations  bit1=0  base1=MSB of Input  bit0=xeng_int_bits  base0=LSB of Input  dbl_ovrd=off  block_type=slice
      settings: infoedit="Extracts a given range of bits from each input sample and presents it at the output.  The output type is ordinarily unsigned with binary point at zero, but can be Boolean when the slice is one bit wide.<P><P>Hardware notes: In hardware this block costs nothing."  block_version=10.1.3
  ant_cnt : xbsIndex_r4/Counter  cnt_type=Free Running  cnt_to=1023  operation=Up  start_count=0  cnt_by_val=1  arith_type=Unsigned  n_bits=xeng_int_bits + n_ants_bits  bin_pt=0  load_pin=off  rst=on  en=on  explicit_period=on  period=1  dbl_ovrd=off  use_behavioral_HDL=off  implementation=Fabric  use_rpm=on  block_type=counter
      settings: xl_area=[0, 0, 0, 0, 0, 0, 0]
  spectr_cnt : Outport  OutDataType=sfix(16)  OutScaling=2^0
  err_cnt : Outport  Port=2  OutDataType=sfix(16)  OutScaling=2^0
  last_missed_ant : Outport  Port=3  OutDataType=sfix(16)  OutScaling=2^0
  wires:
  Logical2/1 -> Counter3/2, Register/2
  rst/1 -> Delay55/1
  valid/1 -> Delay1/1
  Counter3/1 -> err_cnt/1
  rcvd/1 -> Delay2/1
  Inverter/1 -> Logical2/2
  Delay55/1 -> Counter3/1, Counter1/1
  Delay1/1 -> Logical2/1, ant_cnt/2
  Delay2/1 -> Inverter/1
  Delay3/1 -> Counter1/2, ant_cnt/1
  Counter1/1 -> spectr_cnt/1
  sync/1 -> Delay3/1
  Register/1 -> last_missed_ant/1
  ant_cnt/1 -> Slice/1
  Slice/1 -> Register/1

== system r_1f_2x_4a_r340/miss_cnt1 ==
  rst : Inport  OutDataType=sfix(16)  OutScaling=2^0
  valid : Inport  Port=2  OutDataType=sfix(16)  OutScaling=2^0
  rcvd : Inport  Port=3  OutDataType=sfix(16)  OutScaling=2^0
  sync : Inport  Port=4  OutDataType=sfix(16)  OutScaling=2^0
  Counter1 : xbsIndex_r4/Counter  cnt_type=Free Running  cnt_to=1023  operation=Up  start_count=0  cnt_by_val=1  arith_type=Unsigned  n_bits=32  bin_pt=0  load_pin=off  rst=on  en=on  explicit_period=on  period=1  dbl_ovrd=off  use_behavioral_HDL=off  implementation=Fabric  use_rpm=on  block_type=counter
      settings: xl_area=[0, 0, 0, 0, 0, 0, 0]
  Counter3 : xbsIndex_r4/Counter  cnt_type=Free Running  cnt_to=1023  operation=Up  start_count=0  cnt_by_val=1  arith_type=Unsigned  n_bits=32  bin_pt=0  load_pin=off  rst=on  en=on  explicit_period=on  period=1  dbl_ovrd=off  use_behavioral_HDL=off  implementation=Fabric  use_rpm=on  block_type=counter
      settings: xl_area=[0, 0, 0, 0, 0, 0, 0]
  Delay{1..3,55} (4 alike) : xbsIndex_r4/Delay  en=off  latency=2  dbl_ovrd=off  reg_retiming=on  block_type=delay
      settings: xl_area=[0, 0, 0, 0, 0, 0, 0]
  Inverter : xbsIndex_r4/Inverter  en=off  latency=0  dbl_ovrd=off  block_type=inv
      settings: xl_area=[0, 0, 0, 0, 0, 0, 0]
  Logical2 : xbsIndex_r4/Logical  logical_function=AND  inputs=2  en=off  latency=0  precision=Full  arith_type=Unsigned  n_bits=8  bin_pt=2  align_bp=on  dbl_ovrd=off  block_type=logical
      settings: xl_area=[0, 0, 0, 0, 0, 0, 0]
  Register : xbsIndex_r4/Register  init=0  rst=off  en=on  dbl_ovrd=off  block_type=register
  Slice : xbsIndex_r4/Slice  nbits=16  boolean_output=off  mode=Two Bit Locations  bit1=0  base1=MSB of Input  bit0=xeng_int_bits  base0=LSB of Input  dbl_ovrd=off  block_type=slice
      settings: infoedit="Extracts a given range of bits from each input sample and presents it at the output.  The output type is ordinarily unsigned with binary point at zero, but can be Boolean when the slice is one bit wide.<P><P>Hardware notes: In hardware this block costs nothing."  block_version=10.1.3
  ant_cnt : xbsIndex_r4/Counter  cnt_type=Free Running  cnt_to=1023  operation=Up  start_count=0  cnt_by_val=1  arith_type=Unsigned  n_bits=xeng_int_bits + n_ants_bits  bin_pt=0  load_pin=off  rst=on  en=on  explicit_period=on  period=1  dbl_ovrd=off  use_behavioral_HDL=off  implementation=Fabric  use_rpm=on  block_type=counter
      settings: xl_area=[0, 0, 0, 0, 0, 0, 0]
  spectr_cnt : Outport  OutDataType=sfix(16)  OutScaling=2^0
  err_cnt : Outport  Port=2  OutDataType=sfix(16)  OutScaling=2^0
  last_missed_ant : Outport  Port=3  OutDataType=sfix(16)  OutScaling=2^0
  wires:
  Slice/1 -> Register/1
  ant_cnt/1 -> Slice/1
  Register/1 -> last_missed_ant/1
  sync/1 -> Delay3/1
  Counter1/1 -> spectr_cnt/1
  Delay3/1 -> ant_cnt/1, Counter1/2
  Delay2/1 -> Inverter/1
  Delay1/1 -> ant_cnt/2, Logical2/1
  Delay55/1 -> Counter1/1, Counter3/1
  Inverter/1 -> Logical2/2
  rcvd/1 -> Delay2/1
  Counter3/1 -> err_cnt/1
  valid/1 -> Delay1/1
  rst/1 -> Delay55/1
  Logical2/1 -> Register/2, Counter3/2
